simulink model "MCVT_Integration"  (R2020a)
settings: Solver=ode8  StartTime=0.0  StopTime=T_end  FixedStep=dt  RelTol=1e-3  EnableMultiTasking=on
values from neighbouring files (symbols this model uses):
  assumed_power_voltage = 1.25e2    (= 125)    [MCVT_Input_File.m]    (also in MCVT_Run_QuickCheck.m)
  battery_power_toggle = 1    [MCVT_Input_File.m]    (also in MCVT_Run_QuickCheck.m)
  battery_trickle_charge_param = 0.1    [MCVT_Input_File.m]    (also in MCVT_Run_QuickCheck.m)
  battery_trickle_leakage_param = 0.01    [MCVT_Input_File.m]    (also in MCVT_Run_QuickCheck.m)
  damage_case_id = 3    [MCVT_Input_File.m]    (also in MCVT_Run_QuickCheck.m = 1)
  dt = 1/fs    (= 0.001)    [MCVT_Run_QuickCheck.m]    (also in MCVT_Sim_Set.m)
  dt_2 = 1/fs_2    (= 0.001)    [MCVT_Run_QuickCheck.m]    (also in MCVT_Sim_Set.m)
  dt_3 = 1/fs_3    (= 0.001)    [MCVT_Run_QuickCheck.m]    (also in MCVT_Sim_Set.m)
  dt_5 = 1/fs_5    (= 0.005)    [MCVT_Run_QuickCheck.m]    (also in MCVT_Sim_Set.m)
  dt_8 = 1/fs_8    (= 0.05)    [MCVT_Run_QuickCheck.m]    (also in MCVT_Sim_Set.m)
  dt_9 = 1/fs_9    (= 0.001)    [MCVT_Run_QuickCheck.m]    (also in MCVT_Sim_Set.m)
  dust_met_duration = 3    [MCVT_Input_File.m]    (also in MCVT_Run_QuickCheck.m = 2)
  ECLSS_indoor_set_temperature = 298.15    [MCVT_Input_File.m]    (also in MCVT_Run_QuickCheck.m)
  energy_store_max_o = 150    [MCVT_Input_File.m]    (also in MCVT_Run_QuickCheck.m)
  energy_store_o = 120    [MCVT_Input_File.m]    (also in MCVT_Run_QuickCheck.m)
  fs = 1000    [MCVT_Run_QuickCheck.m]    (also in MCVT_Sim_Set.m)
  gain_charge_param = 1    [MCVT_Input_File.m]    (also in MCVT_Run_QuickCheck.m)
  ground_temperature = 373    [MCVT_Habitat_Design_Input_File.m]    (also in MCVT_Run_QuickCheck.m)
  I_max_charge = 800    [MCVT_Input_File.m]    (also in MCVT_Run_QuickCheck.m)
  I_max_discharge = 800    [MCVT_Input_File.m]    (also in MCVT_Run_QuickCheck.m)
  initial_pressure = 1e5    (= 100000)    [MCVT_Input_File.m]    (also in MCVT_Run_QuickCheck.m)
  initial_temperature = 295    [MCVT_Input_File.m]    (also in MCVT_Run_QuickCheck.m)
  interior_leak_rate = 0.00415    [MCVT_Input_File.m]    (also in MCVT_Run_QuickCheck.m)
  launch_dust_accum_start_time = 10    [MCVT_Input_File.m]    (also in MCVT_Run_QuickCheck.m = 2)
  launch_dust_accum_stop_time = 12    [MCVT_Input_File.m]    (also in MCVT_Run_QuickCheck.m = 3)
  meteorite_impact_time = 2    [MCVT_Input_File.m]    (also in MCVT_Run_QuickCheck.m = 1)
  nuclear_power_toggle = 1    [MCVT_Input_File.m]    (also in MCVT_Run_QuickCheck.m)
  print_interval = 30    [MCVT_Input_File.m]
  SOC_lower_thres = 0.5    [MCVT_Input_File.m]    (also in MCVT_Run_QuickCheck.m)
  SOC_upper_thres = 0.9    [MCVT_Input_File.m]    (also in MCVT_Run_QuickCheck.m)
  solar_power_toggle = 1    [MCVT_Input_File.m]    (also in MCVT_Run_QuickCheck.m)
  space_temperature = 4    [MCVT_Habitat_Design_Input_File.m]    (also in MCVT_Run_QuickCheck.m)
  Start_angle = 90    [MCVT_Input_File.m]    (also in MCVT_Run_QuickCheck.m)
undefined symbols (not defined in the file or in its folder): A_flow_restrictor, ACTIVITY_LOOKUP_TABLE, air, Aref, Bmap, Bmap2, Bmap3, comms, DiscIntNeverNeededParam, DmgSPL, dust_parameters, ECLSS_dust_damage_repair_time, ECLSS_heater_capacity, ECLSS_IPS_restrictor, ECLSS_IPS_setpointPressure, ECLSS_paint_damage_repair_time, ECLSS_radiatorLoop_discreteNumber, ECLSS_Vst, emptyActivity, expected_heat_add, FI, habitat, HIEM2SMM, HIEM2STM, HIEMDamageElements, impact_numbering, initial_SPLT_damage_index, input_air, input_Cond, input_CP, input_discretization, input_EV, input_Evap, input_radiatorLoop, K_ECLSS_dust_single, K_ECLSS_paint_single, K_Structure_damage_single, Kref, kW_to_W, meteoroidDustDep, mFRF, ndelays, nominalDustDep, noverlap, nuclear_toggle_gain, output_limits, panel_paint0, Ps0, reference_temperature, RepairTime_ECLSS_factor, Sensor_Location_SMM, SMM_T0, SMM_T1, SMM_T2, SMM_T3, SMM_T4, SMM_T4_0, SMM_T4_1, SMM_T4_2, solar_FDD_K ... (+73)
libraries: neural x350, simulink x39, xpcutilitieslib x7, xpcrtudplib x7, dspstat3 x1
(part 42 of 43)

== matlab function MCVT_Integration/Sys8 - Interior Environment/Habitat Interior Environment Model/AirPressureDistribution ==
  data: PrevAirPressure input; Time input; IPCS_Supply input; IPCS_Discharge input; IEN_ADJ_HIEM input; Damage input; HIEMDamageElements input; AirPressure output; IPCSAirflow output; temp0 input; pres0 input; interior_leak_rate input
function [AirPressure,IPCSAirflow] = AirPressureDistribution(PrevAirPressure,Time,IPCS_Supply,IPCS_Discharge,IEN_ADJ_HIEM,Damage,HIEMDamageElements,temp0,pres0,interior_leak_rate)
%% Documentation
% Purpose: To calculate the pressure distribution of the interior air based
%          on interactions with the STM, SMM, and ECLSS models. For
%          more details see Chapter 7 of the documentation report,
%          henceforth referred to as "report".
% Date Created: 3 June 2021
% Date Last Modified: 7 September 2021
% Modeler Name: Jeffrey Steiner (UConn)
% Funding Acknowledgement: Funded by the NASA RETHi Project (Grant #80NSSC19K1076)

% Version Number: MCVT v5

% Subsystem Connections: This code receives inputs from Subsystems 2 
%     and 5. This code ultimately sends outputs to the same subsystems, as
%     well as to Subsystem 1.

% INPUTS %
%   - PrevAirPressure: Double: previous pressure values from memory, 112x1
%       (Pa)
%   - Time: Double: Constant pertaining to simulation time used for logical
%       determinations and application of initial conditions
%   - IPCS_Supply: Double: Constant supplied by ECLSS that represents mass
%       flow of air being added to the system by the Pressure Control(kg/s)
%   - IPCS_Discharge: Double: Constant supplied by ECLSS that represents mass
%       flow of air being removed from the system by the Pressure Control(kg/s)
%   - IEN_ADJ_HIEM: Double: 112x7 matrix with columns detailing the zone 
%       number for the North, South, East, West, Top, and Bottom faces of each
%       zone, with row 1 being the number of the zone of interest
%   - Damage: Double: number of damaged nodes as given by the SMM
%   - HIEMDamageElements: Double: Vector of zone numbers corrseponding to
%       potentially damaged nodes according to mapping from the SMM
%   - temp0: Double: User-Defined initial temperature, constant (K)
%   - pres0: Double: User-Defined initial pressure, constant (K)
%   - interior_leak_rate: Double: User defined leak rate
    
% OUTPUTS %
%   - AirPressure: Double: 112x1 matrix representing the Air Pressure in
%       each corresponding zone (Pa)
%   - IPCSAirflow: Double: Constant representing discharge mass flow to the
%       ECLSS for purposes of air circulation (kg/s)

% No Function Dependencies

% No Data Dependencies

%% System Parameter Definitions
PressureDam = 0; % Exterior Environment Vacuum, Pa
NumElem = 112; % Number of finite elements
MaxIter = 1000; % Maximum number of iterations
n1 = 0.5; % Exponent for Large Openings
F = 0.83; % PowerLaw Flow Coefficient
Pinit = pres0; % Initial Pressure, Pa
Tinit = temp0; % Initial Temperature, K
PCellgsm = ones(NumElem,MaxIter)*100000; % Pressure Iteration Variable, Pa
Mu = 0.00001825;    %Air Viscosity (constant for now), kg/m-s
PCell = zeros(NumElem,2); % Pressure Reporting Variable, Pa
Rind = 287.05; %Individual Gas Constant, J/kg-K
R = 8314.41; % Universal Gas Constant, N-m/kg-mol-K
M = 28.9645; % Mean Molecular Weight per Mole, kg/kg-mole
RhoAir0 = (M*(Pinit))/(R * Tinit); %Initial Air Density, kg/m^3

%% Define Indexed Variables for Simulink

% Laminar Flow Coefficient Variables
Clf = zeros(NumElem,1);
DeltaPtrans = zeros(NumElem,1);
Ck = zeros(NumElem,1);

% Directional Mass Flows
MEast = zeros(NumElem,100);
MWest = zeros(NumElem,100);
MNorth = zeros(NumElem,100);
MSouth = zeros(NumElem,100);
MTop = zeros(NumElem,100);
MBottom = zeros(NumElem,100);

% Directional Mass Flow Derivatives
dMdPEast = zeros(NumElem,100);
dMdPWest = zeros(NumElem,100);
dMdPNorth = zeros(NumElem,100);
dMdPSouth = zeros(NumElem,100);
dMdPTop = zeros(NumElem,100);
dMdPBottom = zeros(NumElem,100);

% Iteration Variables
MCellgsm = zeros(NumElem,100);
PDeriv = zeros(NumElem,100);

% Damaged Elements as given by the SMM
Damaged = zeros(NumElem,1);

% Application of Initial Conditions
if Time == -1 || Time == 1
    PrevAirPressure = ones(NumElem,1)*Pinit;
end

if Time == -1
    for el = 1:NumElem
        PCellgsm(el,1) = Pinit;
    end
else
    PCellgsm(:,1) = PrevAirPressure;
end

%% Begin Calculation Loop

for PInc = 1

    % Damage Parameter determination, used to find leak locations
    DamagedNodes = find(Damage > 0);
    
    for checkDam = 1:length(DamagedNodes)
        Damaged(checkDam) = HIEMDamageElements(DamagedNodes(checkDam));
    end
    DamagedNZ = nonzeros(Damaged);
    DamagedElements = unique(DamagedNZ);
    
    %% System Inputs and Outputs
    
    Input1 = IPCS_Supply;
    Output1 = -(IPCS_Discharge*(Pinit/(Rind*temp0)));
    
    Inputs = [Input1];
    
    BC_SupplyFlow = Inputs(1); %kg/s
    BC_DischargeFlow = Output1; %kg/s
    
    PBC_Supply = PCellgsm(12,1) + ((BC_SupplyFlow^2)/F);
    PBC_Discharge = PCellgsm(50,1) - ((BC_DischargeFlow^2)/F);
    
    % Initiation of "While" Loop
    Condition = ones(NumElem,1);
    gsm = 1;
    
    while Condition(Condition >= 0.5)
        
        % Calculation of Mass Flows
        for Elem = 1:NumElem
            for j = 2
                if (IEN_ADJ_HIEM(j,Elem)) == 0
                    if Elem == 50
                        MEast(Elem,gsm) = F * sqrt(abs(PCellgsm(Elem,gsm) - PBC_Discharge));
                        dMdPEast(Elem,gsm) = -F * n1 / abs(PCellgsm(Elem,gsm) - PBC_Discharge);
                    else
                        MEast(Elem,gsm) = 0;
                        dMdPEast(Elem,gsm) = 0;
                    end
                    
                else
                    if PCellgsm(IEN_ADJ_HIEM(j,Elem),gsm) > PCellgsm(Elem,gsm)
                        MEast(Elem,gsm) = -F * sqrt(abs(PCellgsm(Elem,gsm) - PCellgsm(IEN_ADJ_HIEM(j,Elem),gsm)));
                        dMdPEast(Elem,gsm) = F * n1 / abs(PCellgsm(Elem,gsm) - PCellgsm(IEN_ADJ_HIEM(j,Elem),gsm));
                    elseif PCellgsm(IEN_ADJ_HIEM(j,Elem),gsm) - PCellgsm(Elem,gsm) == 0
                        dMdPEast(Elem,gsm) = 0;
                        MEast(Elem,gsm) = 0;
                    else
                        MEast(Elem,gsm) = F * sqrt(abs(PCellgsm(Elem,gsm) - PCellgsm(IEN_ADJ_HIEM(j,Elem),gsm)));
                        dMdPEast(Elem,gsm) = -F * n1 / abs(PCellgsm(Elem,gsm) - PCellgsm(IEN_ADJ_HIEM(j,Elem),gsm));
                    end
                end
            end
            for j = 3
                if (IEN_ADJ_HIEM(j,Elem)) == 0
                    if Elem == 12
                        MWest(Elem,gsm) = -F * sqrt(abs(PCellgsm(Elem,gsm) - PBC_Supply));
                        dMdPWest(Elem,gsm) = F * n1 / abs(PCellgsm(Elem,gsm) - PBC_Supply);
                    else
                        MWest(Elem,gsm) = 0;
                        dMdPWest(Elem,gsm) = 0;
                    end
                else
                    if PCellgsm(IEN_ADJ_HIEM(j,Elem),gsm) > PCellgsm(Elem,gsm)
                        MWest(Elem,gsm) = -F * sqrt(abs(PCellgsm(Elem,gsm) - PCellgsm(IEN_ADJ_HIEM(j,Elem),gsm)));
                        dMdPWest(Elem,gsm) = F * n1 / abs(PCellgsm(Elem,gsm) - PCellgsm(IEN_ADJ_HIEM(j,Elem),gsm));
                    elseif PCellgsm(IEN_ADJ_HIEM(j,Elem),gsm) - PCellgsm(Elem,gsm) == 0
                        dMdPWest(Elem,gsm) = 0;
                        MWest(Elem,gsm) = 0;
                    else
                        MWest(Elem,gsm) = F * sqrt(abs(PCellgsm(Elem,gsm) - PCellgsm(IEN_ADJ_HIEM(j,Elem),gsm)));
                        dMdPWest(Elem,gsm) = -F * n1 / abs(PCellgsm(Elem,gsm) - PCellgsm(IEN_ADJ_HIEM(j,Elem),gsm));
                    end
                end
            end
            for j = 4
                if (IEN_ADJ_HIEM(j,Elem)) == 0
                    if Elem == 1
                        MNorth(Elem,gsm) = 0;
                        dMdPNorth(Elem,gsm) = 0;
                    elseif Elem == 2
                        MNorth(Elem,gsm) = 0;
                        dMdPNorth(Elem,gsm) = 0;
                    else
                        MNorth(Elem,gsm) = 0;
                        dMdPNorth(Elem,gsm) = 0;
                    end
                else
                    if PCellgsm(IEN_ADJ_HIEM(j,Elem),gsm) > PCellgsm(Elem,gsm)
                        MNorth(Elem,gsm) = -F * sqrt(abs(PCellgsm(Elem,gsm) - PCellgsm(IEN_ADJ_HIEM(j,Elem),gsm)));
                        dMdPNorth(Elem,gsm) = F * n1 / abs(PCellgsm(Elem,gsm) - PCellgsm(IEN_ADJ_HIEM(j,Elem),gsm));
                    elseif PCellgsm(IEN_ADJ_HIEM(j,Elem),gsm) - PCellgsm(Elem,gsm) == 0
                        dMdPNorth(Elem,gsm) = 0;
                        MNorth(Elem,gsm) = 0;
                    else
                        MNorth(Elem,gsm) = F * sqrt(abs(PCellgsm(Elem,gsm) - PCellgsm(IEN_ADJ_HIEM(j,Elem),gsm)));
                        dMdPNorth(Elem,gsm) = -F * n1 / abs(PCellgsm(Elem,gsm) - PCellgsm(IEN_ADJ_HIEM(j,Elem),gsm));
                    end
                end
            end
            for j = 5
                if (IEN_ADJ_HIEM(j,Elem)) == 0
                    if Elem == 1
                        MSouth(Elem,gsm) = 0;
                        dMdPSouth(Elem,gsm) = 0;
                    elseif Elem == 2
                        MSouth(Elem,gsm) = 0;
                        dMdPSouth(Elem,gsm) = 0;
                    else
                        MSouth(Elem,gsm) = 0;
                        dMdPSouth(Elem,gsm) = 0;
                    end
                    
                else
                    if PCellgsm(IEN_ADJ_HIEM(j,Elem),gsm) > PCellgsm(Elem,gsm)
                        MSouth(Elem,gsm) = -F * sqrt(abs(PCellgsm(Elem,gsm) - PCellgsm(IEN_ADJ_HIEM(j,Elem),gsm)));
                        dMdPSouth(Elem,gsm) = F * n1 / abs(PCellgsm(Elem,gsm) - PCellgsm(IEN_ADJ_HIEM(j,Elem),gsm));
                    elseif PCellgsm(IEN_ADJ_HIEM(j,Elem),gsm) - PCellgsm(Elem,gsm) == 0
                        dMdPSouth(Elem,gsm) = 0;
                        MSouth(Elem,gsm) = 0;
                    else
                        MSouth(Elem,gsm) = F * sqrt(abs(PCellgsm(Elem,gsm) - PCellgsm(IEN_ADJ_HIEM(j,Elem),gsm)));
                        dMdPSouth(Elem,gsm) = -F * n1 / abs(PCellgsm(Elem,gsm) - PCellgsm(IEN_ADJ_HIEM(j,Elem),gsm));
                    end
                end
            end
            for j = 6
                if (IEN_ADJ_HIEM(j,Elem)) == 0
                    MTop(Elem,gsm) = 0;
                    dMdPTop(Elem,gsm) = 0;
                else
                    if PCellgsm(IEN_ADJ_HIEM(j,Elem),gsm) > PCellgsm(Elem,gsm)
                        MTop(Elem,gsm) = -F * sqrt(abs(PCellgsm(Elem,gsm) - PCellgsm(IEN_ADJ_HIEM(j,Elem),gsm)));
                        dMdPTop(Elem,gsm) = F * n1 / abs(PCellgsm(Elem,gsm) - PCellgsm(IEN_ADJ_HIEM(j,Elem),gsm));
                    elseif PCellgsm(IEN_ADJ_HIEM(j,Elem),gsm) - PCellgsm(Elem,gsm) == 0
                        dMdPTop(Elem,gsm) = 0;
                        MSouth(Elem,gsm) = 0;
                    else
                        MTop(Elem,gsm) = F * sqrt(abs(PCellgsm(Elem,gsm) - PCellgsm(IEN_ADJ_HIEM(j,Elem),gsm)));
                        dMdPTop(Elem,gsm) = -F * n1 / abs(PCellgsm(Elem,gsm) - PCellgsm(IEN_ADJ_HIEM(j,Elem),gsm));
                    end
                end
            end
            for j = 7
                if (IEN_ADJ_HIEM(j,Elem)) == 0
                    MBottom(Elem,gsm) = 0;
                    dMdPBottom(Elem,gsm) = 0;
                else
                    if PCellgsm(IEN_ADJ_HIEM(j,Elem),gsm) > PCellgsm(Elem,gsm)
                        MBottom(Elem,gsm) = -F * sqrt(abs(PCellgsm(Elem,gsm) - PCellgsm(IEN_ADJ_HIEM(j,Elem),gsm)));
                        dMdPBottom(Elem,gsm) = F * n1 / abs(PCellgsm(Elem,gsm) - PCellgsm(IEN_ADJ_HIEM(j,Elem),gsm));
                    elseif PCellgsm(IEN_ADJ_HIEM(j,Elem),gsm) - PCellgsm(Elem,gsm) == 0
                        dMdPBottom(Elem,gsm) = 0;
                        MSouth(Elem,gsm) = 0;
                    else
                        MBottom(Elem,gsm) = F * sqrt(abs(PCellgsm(Elem,gsm) - PCellgsm(IEN_ADJ_HIEM(j,Elem),gsm)));
                        dMdPBottom(Elem,gsm) = -F * n1 / abs(PCellgsm(Elem,gsm) - PCellgsm(IEN_ADJ_HIEM(j,Elem),gsm));
                    end
                end
            end
            
        end
        
        % Compilation of calculated vectors and derivatives for easy
        % code-level analysis        
        % Mass Flow Vectors
        East = MEast(:,gsm)';
        West = MWest(:,gsm)';
        North = MNorth(:,gsm)';
        South = MSouth(:,gsm)';
        Top = MTop(:,gsm)';
        Bottom = MBottom(:,gsm)';
        MassFlows = [East; West; North; South; Top; Bottom];
        % Mass Flow Derivatives
        dEast = dMdPEast(:,gsm)';
        dWest = dMdPWest(:,gsm)';
        dNorth = dMdPNorth(:,gsm)';
        dSouth = dMdPSouth(:,gsm)';
        dTop = dMdPTop(:,gsm)';
        dBottom = dMdPBottom(:,gsm)';
        dMassFlows = [dEast; dWest; dNorth; dSouth; dTop; dBottom];
        
        MCellgsm(:,gsm) = MEast(:,gsm) + MWest(:,gsm) + MNorth(:,gsm) + MSouth(:,gsm) + MTop(:,gsm) + MBottom(:,gsm);
        
        % Add in Damage components to account for leaks
        for checkDam = 1:NumElem
            for daminc = 1:length(DamagedElements)
                if checkDam == (DamagedElements(daminc))
                    BCDamFlow = interior_leak_rate * sqrt(abs(PCellgsm(checkDam,1) - PressureDam));
                    MCellgsm(checkDam,gsm) = MCellgsm(checkDam,gsm) + BCDamFlow;
                else
                    continue
                end
            end
        end
        
        PDeriv(:,gsm) = (dMdPNorth(:,gsm) + dMdPSouth(:,gsm) + dMdPWest(:,gsm) + dMdPEast(:,gsm) + dMdPTop(:,gsm) + dMdPBottom(:,gsm));
        
        % Mass Residual
        B = MCellgsm(:,gsm);
        
        dx = (32.725/NumElem)^(1/3);
        dy = (32.725/NumElem)^(1/3);
        
        % Calculation of Laminar Flow Coefficient
        Re = 30;
        Area = dx * dy;
        Dlf = (4*Area)/(4*dx);
        Ftrans = (Mu*Re*Area)/Dlf;
        
        for count = 1:NumElem
            Clf(count) = (0.6*Area)/sqrt(RhoAir0/2);
            DeltaPtrans(count) = (Ftrans/Clf(count))^2;
            Ck(count) = (Mu*Ftrans)/(RhoAir0*DeltaPtrans(count));
        end
        
        %Assemble Jacobian Matrix
        JCB = zeros(NumElem);
        
        for JCBi = 1:NumElem
            
            A=IEN_ADJ_HIEM(2:end,JCBi);
            NZ=A(A~=0);
            for k = 1:NumElem
                if k==JCBi
                    JCB(JCBi,k) = 1;
                elseif k == NZ(NZ == k)
                    if k < JCBi
                        JCB(JCBi,k) = JCB(k,JCBi);
                    else
                        if (PCellgsm(k,gsm)-PCellgsm(JCBi,gsm)) == 0
                            JCB(JCBi,k) = F * n1 * (0.0001^-n1);
                        else
                            JCB(JCBi,k) = ((-F * n1)/sqrt(abs((PCellgsm(k,gsm)-PCellgsm(JCBi,gsm)))));
                        end
                    end
                else
                    JCB(JCBi,k) = 0;
                end
            end
            if JCBi == 12
                DiagSum = [1:(JCBi-1) (JCBi+1):NumElem];
                JCB(JCBi,JCBi) = sum(abs(JCB(JCBi,DiagSum))) + abs(((-F * n1)/sqrt(abs((PCellgsm(JCBi,gsm)-PBC_Supply)))));

            elseif JCBi == 50
                DiagSum = [1:(JCBi-1) (JCBi+1):NumElem];
                JCB(JCBi,JCBi) = sum(abs(JCB(JCBi,DiagSum))) + abs(((-F * n1)/sqrt(abs((PCellgsm(JCBi,gsm)-PBC_Discharge)))));
            else
                if JCBi == 1
                    DiagSum = [(JCBi+1):NumElem];
                else
                    DiagSum = [1:(JCBi-1) (JCBi+1):NumElem];
                end
                JCB(JCBi,JCBi) = sum(abs(JCB(JCBi,DiagSum)));
            end
        end
        
        % Determine Pressure Correction Vector
        C = zeros(NumElem,1);
        C(:,1) = B'/JCB;
        
        % Update Pressure Vector
        PCellgsm(:,gsm+1) = PCellgsm(:,gsm) - C;
        
        % Establish value of convergence check
        Condition = abs(PCellgsm(:,gsm+1)-PCellgsm(:,gsm));
        
        % Increase increment value
        gsm = gsm+1;
        
    end
    
    % Override to prevent negative pressure values in case of pressure
    % reductions
    if PCellgsm(PCellgsm < 0) < 0
        PCellgsm(PCellgsm < 0) = 0;
    end
    
    % Update pressure recording variable
    PCell(:,PInc+1) = PCellgsm(:,gsm);
end

%% Output Initialization and Execution

AirPressure = squeeze(PCell(:,2)); % Air Pressure Distribution, Pa
IPCSAirflow = IPCS_Discharge; % Airflow to ECLSS, kg/s

end

== matlab function MCVT_Integration/Sys8 - Interior Environment/Habitat Interior Environment Model/AirTemperatureDistribution ==
  data: PCell0 input; HIEMDamageElements input; RefTemp input; pres0 input; temp0 input; Damage input; HCAirFlow input; WallTemp input; AirTemp output; ThermalManHeatingInp input; ThermalManHeatingInpLoc input; ThermalManCoolingInp input; ThermalManCoolingInpLoc input; AirTempWall output; Time input; PSTemp output size=1; dt input; IEN_ADJ_HIEM input; HumanHeat input; HumanElement input; TMSAirflow output; air_specific_heat input; habitat_volume input
function [AirTemp,AirTempWall,PSTemp,TMSAirflow] = TemperatureDistribution3D(PCell0,HIEMDamageElements,RefTemp,pres0,temp0,Damage,HCAirFlow,WallTemp,ThermalManHeatingInp,ThermalManHeatingInpLoc,ThermalManCoolingInp,ThermalManCoolingInpLoc,Time,dt,IEN_ADJ_HIEM,HumanHeat,HumanElement,air_specific_heat,habitat_volume)
%% Documentation
% Purpose: To calculate the temperature distribution of the interior air based
%          on interactions with the STM, SMM, Human, and ECLSS models. For
%          more details see Chapter 7 of the documentation report,
%          henceforth referred to as "report".
% Date Created: 3 June 2021
% Date Last Modified: 7 September 2021
% Modeler Name: Jeffrey Steiner (UConn)
% Funding Acknowledgement: Funded by the NASA RETHi Project (Grant #80NSSC19K1076)

% Version Number: MCVT v5

% Subsystem Connections: This code receives inputs from Subsystems 2, 5, 6,
%     and 9. This code sends outputs to Subsystems 1, 2, 5, and 6.

% INPUTS %
%   - PCell0: Double: Pressure Distribution, Pa
%   - HIEMDamageElements: Double: Vector of zone numbers corresponding to
%       potentially damaged nodes according to mapping from the SMM
%   - RefTemp: Double: User-Defined Reference Temperature for the Exterior
%   Environment in case of leak scenario (K)
%   - pres0: Double: User-Defined initial pressure, constant (Pa)
%   - temp0: Double: User-Defined initial temperature, constant (K)
%   - Damage: Double: number of damaged nodes as given by the SMM
%   - HCAirFlow: Double: Constant supplied by ECLSS that represents mass
%       flow of air being added to the system from the temperature control(kg/s)
%   - WallTemp: Double: Mapped interior wall surface temperature from STM,
%       64x1 (K)
%   - ThermalManHeatingInp: Double: Constant Total Heating Energy Input 
%     from ECLSS Thermal Management (J) 
%   - ThermalManHeatingInpLoc: Double: Zone number corresponding to heating
%       input location for ECLSS Thermal Management
%   - ThermalManCoolingInp: Double: Constant Cooling Temperature Setpoint
%     Input from ECLSS Thermal Management (K) 
%   - ThermalManCoolingInpLoc: Double: Zone number corresponding to cooling
%       input location for ECLSS Thermal Management
%   - Time: Double: Constant pertaining to simulation time used for logical
%       determinations and application of initial conditions
%   - dt: Double: constant representing the time step used for the model
%       (s)
%   - IEN_ADJ_HIEM: Double: 112x7 matrix with columns detailing the zone 
%       number for the North, South, East, West, Top, and Bottom faces of each
%       zone, with row 1 being the number of the zone of interest
%   - HumanHeat: Double: Constant Total Heating Energy Input from Human 
%       Agent Model (J) 
%   - HumanElement: Double: Zone number corresponding to heating input
%       location for Human Agent Model
%   - air_specific_heat: Double: Specific Heat of Air, J/kg-K
%   - habitat_volume: Double: User-defined habitat volume, m^3
    
% OUTPUTS %
%   - AirTemp: Double: 112x1 matrix representing the Air Temperature in
%       each corresponding zone (K)
%   - AirTempWall: 20x1 matrix corresponding to the Air Temperature in
%       zones in contact with the structural wall (K), sent to STM
%   - PSTemp: Double: Constant Zonal Temperature corresponding to zone in
%       which ECLSS Cooling input is provided (K), provided to ECLSS Thermal
%       Management
%   - TMSAirflow: Double: Constant representing discharge mass flow to the
%       ECLSS Thermal Management for purposes of air circulation (kg/s)

% No Function Dependencies

% No Data Dependencies

%% System Parameter Definitions

NumElem = length(IEN_ADJ_HIEM); % Number of Elements in the system
TCell0 = temp0; %Initial Air Temperature, K
Rind = 287.05; %Individual Gas Constant, J/kg-K
WallElements = [1;2;3;4;5;6;7;8;9;10;11;12;13;14;15;16;29;30;31;32;45;46;47;48;49;50;51;52;53;54;55;56;57;58;59;60;61;62;75;76;77;78;79;80;81;82;83;84;97;98;99;100;101;102;103;104;105;106;107;108;109;110;111;112];
h = 10;
WallElemArea = ((habitat_volume * 3)/2.5)/length(WallElements);
TBS = 293.15;
%% Define Indexed Variables for Simulink

% Directional Energy Flows
QEast = zeros(NumElem,1);
QWest = zeros(NumElem,1);
QNorth = zeros(NumElem,1);
QSouth = zeros(NumElem,1);
QTop = zeros(NumElem,1);
QBottom = zeros(NumElem,1);
QCell = zeros(NumElem,1);

RhoAir0 = pres0/(Rind * temp0); %Initial Air Density, kg/m^3

% Heat Conduction Coefficient
Sys8_CondCoeff = zeros(NumElem,1);

% Heat Change Iteration Variable
dTCell = zeros(NumElem,1);

% Damaged Elements as given by the SMM
Damaged = zeros(NumElem,1);

% Temeprature Recording Variable
TCell = ones(NumElem,2);

if Time == -1
    for el = 1:NumElem
        TCell(el,1) = TCell0;
    end
    MassFlows = zeros(6,NumElem);
else
    TCell(:,1) = PCell0(:,1)/(RhoAir0*Rind);
end

%% Definition of Wall Boundary Condition

% Set Cooling input to 0 if no airflow is provided
if HCAirFlow == 0
    ThermalManCoolingInp = 0;
end

%% Temperature Calculation Loop Initialization

% Zonal Density Variable
RhoAir0 = zeros(NumElem,1);
RhoAir0(:,1) = PCell0(:,1)/(Rind * TCell0); %Initial Air Density, kg/m^3

Sys8_AirCond = 0.025; %Thermal Conductivity of air, W/m-K
Sys8_CondCoeff(:,1) = (Sys8_AirCond./(RhoAir0(:,1)*air_specific_heat)); % Thermal Conduction Coefficient

% Loop calculating temperature change from Heating/Cooling as received by
% ECLSS, K
if HCAirFlow == 0
    CoolingInp = 0;
    HeatingInp = ((ThermalManHeatingInp/(air_specific_heat * RhoAir0(ThermalManHeatingInpLoc,1) * 1))*dt);
else
    CoolingInp = (air_specific_heat * (HCAirFlow * RhoAir0(ThermalManCoolingInpLoc,1)) * (ThermalManCoolingInp - TCell(ThermalManCoolingInpLoc,1))/(air_specific_heat * RhoAir0(ThermalManCoolingInpLoc,1) * 1))*dt;
    HeatingInp = ((ThermalManHeatingInp/(air_specific_heat * RhoAir0(ThermalManHeatingInpLoc,1) * 1))*dt);
end

%% Begin Calculation Loop

for Time = 1
    %% System Inputs and Outputs
    
    % Initiation of "While" Loop
    Condition = ones(NumElem,1);
    
    while Condition(Condition >= 0.5)
        WallElementCount = 1;
        % Calculation of Directional Energy Balances
        for Elem = 1:NumElem
            for j = 2
                if (IEN_ADJ_HIEM(j,Elem)) == 0
                    QEast(Elem,1) = (((Sys8_CondCoeff(Elem,1) * (WallTemp(WallElementCount,1) - TCell(Elem,1)))*(air_specific_heat * RhoAir0(Elem,1) * (sqrt(WallElemArea)/2))) + ((h * WallElemArea * (WallTemp(WallElementCount,1) - TCell(Elem,1)))/(air_specific_heat * RhoAir0(Elem,1) * (habitat_volume/NumElem))));
                end
            end
            for j = 3
                if (IEN_ADJ_HIEM(j,Elem)) == 0
                    QWest(Elem,1) = (((Sys8_CondCoeff(Elem,1) * (WallTemp(WallElementCount,1) - TCell(Elem,1)))*(air_specific_heat * RhoAir0(Elem,1) * (sqrt(WallElemArea)/2))) + ((h * WallElemArea * (WallTemp(WallElementCount,1) - TCell(Elem,1)))/(air_specific_heat * RhoAir0(Elem,1) * (habitat_volume/NumElem))));
                end
            end
            for j = 4
                if (IEN_ADJ_HIEM(j,Elem)) == 0
                    QNorth(Elem,1) = (((Sys8_CondCoeff(Elem,1) * (WallTemp(WallElementCount,1) - TCell(Elem,1)))*(air_specific_heat * RhoAir0(Elem,1) * (sqrt(WallElemArea)/2))) + ((h * WallElemArea * (WallTemp(WallElementCount,1) - TCell(Elem,1)))/(air_specific_heat * RhoAir0(Elem,1) * (habitat_volume/NumElem))));
                end
            end
            for j = 5
                if (IEN_ADJ_HIEM(j,Elem)) == 0
                    QSouth(Elem,1) = (((Sys8_CondCoeff(Elem,1) * (WallTemp(WallElementCount,1) - TCell(Elem,1)))*(air_specific_heat * RhoAir0(Elem,1) * (sqrt(WallElemArea)/2))) + ((h * WallElemArea * (WallTemp(WallElementCount,1) - TCell(Elem,1)))/(air_specific_heat * RhoAir0(Elem,1) * (habitat_volume/NumElem))));
                end
            end
            for j = 6
                if (IEN_ADJ_HIEM(j,Elem)) == 0
                    QTop(Elem,1) = (((Sys8_CondCoeff(Elem,1) * (WallTemp(WallElementCount,1) - TCell(Elem,1)))*(air_specific_heat * RhoAir0(Elem,1) * (sqrt(WallElemArea)/2))) + ((h * WallElemArea * (WallTemp(WallElementCount,1) - TCell(Elem,1)))/(air_specific_heat * RhoAir0(Elem,1) * (habitat_volume/NumElem))));
                end
            end
            for j = 7
                if (IEN_ADJ_HIEM(j,Elem)) == 0
                    QBottom(Elem,1) = (((Sys8_CondCoeff(Elem,1) * (TBS - TCell(Elem,1)))*(air_specific_heat * RhoAir0(Elem,1) * (sqrt(WallElemArea)/2))) + ((h * WallElemArea * (TBS - TCell(Elem,1)))/(air_specific_heat * RhoAir0(Elem,1) * (habitat_volume/NumElem))));
                end
            end
            
            % Increase wall element count - used for sequential application
            % of wall temperature from STM
            if IEN_ADJ_HIEM(IEN_ADJ_HIEM(2:6,Elem) == 0)
                WallElementCount = WallElementCount + 1;
            end
        end
        
        % Compilation of calculated vectors for easy code-level analysis
        % Energy Flow Vectors
        East = QEast(:,1)';
        West = QWest(:,1)';
        North = QNorth(:,1)';
        South = QSouth(:,1)';
        Top = QTop(:,1)';
        Bottom = QBottom(:,1)';
        EnergyFlows = [East; West; North; South; Top; Bottom];
        
        QCell(:,1) = QEast(:,1) + QWest(:,1) + QNorth(:,1) + QSouth(:,1) + QTop(:,1) + QBottom(:,1);
        
        % Consider ECLSS Input
        
        QCell(ThermalManHeatingInpLoc,1) = QCell(ThermalManHeatingInpLoc,1) + HeatingInp;
        QCell(ThermalManCoolingInpLoc,1) = QCell(ThermalManCoolingInpLoc,1) + CoolingInp;
        
        % Consider Damage Input
        
        DamagedNodes = find(Damage > 0);
        
        for checkDam = 1:length(DamagedNodes)
            Damaged(checkDam) = HIEMDamageElements(DamagedNodes(checkDam));
        end
        DamagedElements = unique(Damaged);
        
        for checkDam = 1:NumElem
            for daminc = 1:length(DamagedElements)
                if checkDam == (DamagedElements(daminc))
                    BCDamHeat = ((-h * WallElemArea * (TCell(checkDam,1) - RefTemp))/(air_specific_heat * RhoAir0(checkDam,1) * (habitat_volume/NumElem)));
                    QCell(checkDam,1) = QCell(checkDam,1) + BCDamHeat;
                else
                    continue
                end
            end
        end
        
        % Determine temperature change
        for Sys8_TempInc = 1:NumElem
            dTCell(Sys8_TempInc,1) = (QCell(Sys8_TempInc,1)*dt)/((RhoAir0(Sys8_TempInc,1)*(habitat_volume/NumElem)) * air_specific_heat);
        end
        
        % Consider Human Input, (Given as a value of Kelvin)
        
        dTCell(HumanElement,1) = dTCell(HumanElement,1) + HumanHeat;
        
        % Apply temperature change
        TCell(:,2) = TCell(:,1) + dTCell(:,1);
        
        % Update convergence condition
        Condition = abs(TCell(:,2) - TCell(:,1));
        
        % Update temperature recording variable for next iteration
        TCell(:,1) = TCell(:,2);
    end    
end
%% Output Initialization and Execution

% Outputs to memory blocks
AirTemp = TCell(:,2);

% Designation of Wall-Adjacent Element Temperature Output
OuterElements = zeros(64,1);
ATW = zeros(64,1);
count = 1;
for check = 1:NumElem
    if IEN_ADJ_HIEM(2,check) == 0
        OuterElements(count) = IEN_ADJ_HIEM(1,check);
        ATW(count) = AirTemp(OuterElements(count),1);
        count = count + 1;
    
    elseif IEN_ADJ_HIEM(3,check) == 0
        OuterElements(count) = IEN_ADJ_HIEM(1,check);
        ATW(count) = AirTemp(OuterElements(count),1);
        count = count + 1;
    
    elseif IEN_ADJ_HIEM(4,check) == 0
        OuterElements(count) = IEN_ADJ_HIEM(1,check);
        ATW(count) = AirTemp(OuterElements(count),1);
        count = count + 1;
    
    elseif IEN_ADJ_HIEM(5,check) == 0
        OuterElements(count) = IEN_ADJ_HIEM(1,check);
        ATW(count) = AirTemp(OuterElements(count),1);
        count = count + 1;
    
    elseif IEN_ADJ_HIEM(6,check) == 0
        OuterElements(count) = IEN_ADJ_HIEM(1,check);
        ATW(count) = AirTemp(OuterElements(count),1);
        count = count + 1;
    end
end

% Final Outputs
TMSAirflow = HCAirFlow; % Airflow Output to ECLSS (Constant)
AirTempWall = ATW(:,1); % Wall-Adjacent temp output (to STM), K
PSTemp = TCell(ThermalManCoolingInpLoc,2); % Reference Temp for ECLSS, K

end

== matlab function MCVT_Integration/Sys2 - Structural System/Sys2_Structural_System/Structural Thermal Model 2.2/STM Temperature Sensors/ Resistance Temperature Detector1/Switch ON//OFF Control Block/Switch OFF/MATLAB Function1 ==
  data: u input; y output; Time input
function y = fcn(u,Time)

if Time>=0
    u = NaN;
end

y = u;

== matlab function MCVT_Integration/Sys5 - ECLSS/Active Thermal Control System (ATCS)/MATLAB Function6 ==
  data: P_request output; Ps input; lim_supply input; Ph output; Qh input
function [ P_request, Ph] = heater_capacity(Ps, lim_supply, Qh )
%% Documentation
% Purpose: Output heating power and power request for next timestep
% Date Created: 20 June 2020
% Date Last Modified: 27 July 2021
% Modeler Name: Jaewon Park (Purdue)
% Funding Acknowledgement: Funded by the NASA RETHi Project (80NSSC19K1076)

% Version Number: MCVT v1.4

% Subsystem Connections: receives input from subsystem 3 and sends output to subsystem 8.

%%% All inputs and outputs to this function are 1 dimensional %%%
% INPUTS %
%   - Ps: power required for heating, unit: kW, type: float
%   - Qh: heating capacity, unit: kW, type: float
%   - lim_supply: limited power supply, unit: kW, type: float
    
% OUTPUTS %
%   - Ph: heating power, unit: kW, type: float
%   - P_request: power supply request, unit: kW, type: float

% Function Dependencies: No dependency

% No Data Depenencies
%% Calculated power
Ph = min([Ps,Qh,lim_supply]);   % output heating power is the minimum of three inputs
P_request = min([Ps,Qh]);       % it will also request power it would have needed 

end

== matlab function MCVT_Integration/Sys5 - ECLSS/Active Thermal Control System (ATCS)/Sensors ==
  data: Tfs input; TTfs output
function TTfs= sensors_HTF(Tfs)
%% Documentation
% Purpose: Simulate sensor errors
% Date Created: 20 June 2020
% Date Last Modified: 27 July 2021
% Modeler Name: Jaewon Park, CJ Pan (Purdue)
% Funding Acknowledgement: Funded by the NASA RETHi Project (80NSSC19K1076)

% Version Number: MCVT v1.4

% Subsystem Connections: No connection.

% INPUTS %
%   - Tfs: temperature reading, unit: C, type: float
    
% OUTPUTS %
%   - TTfs: temperature reading with noise, unit: C, type: float

% Function Dependencies: No dependency

% No Data Depenencies
%% adding noise (to do)
N=length(Tfs);

n=zeros(1,N);


for i=1:N
    n(i)=normrnd(0,0.1);
end

TTfs=Tfs+n;


end

== matlab function MCVT_Integration/Sys5 - ECLSS/Active Thermal Control System (ATCS)/Sensors1 ==
  data: Trs input; TTrs output
function TTrs= sensors_rfin(Trs)
%% Documentation
% Purpose: Simulate sensor errors
% Date Created: 20 June 2020
% Date Last Modified: 27 July 2021
% Modeler Name: Jaewon Park, CJ Pan (Purdue)
% Funding Acknowledgement: Funded by the NASA RETHi Project (80NSSC19K1076)

% Version Number: MCVT v1.4

% Subsystem Connections: No connection.

% INPUTS %
%   - Tfs: temperature reading, unit: C, type: float
    
% OUTPUTS %
%   - TTfs: temperature reading with noise, unit: C, type: float

% Function Dependencies: No dependency

% No Data Depenencies
%% adding noise (to do)

N=length(Trs);

n=zeros(1,N);


for i=1:N
    n(i)=normrnd(0,0.1);
end

TTrs=Trs+n;


end

== matlab function MCVT_Integration/Sys5 - ECLSS/Active Thermal Control System (ATCS)/Variable set temperature ==
  data: Pd input; Tset0 input; Ps input; Tset output; Tset input; set input; set output
function [Tset ,set] =set_temperature_change(Pd, Tset0, Ps,  Tset, set)
%% Documentation
% Purpose: Variable temperature setpoint adjustment. When there is less
% power available than the amount needed, setpoint temperature is raised little bit 
% so that refridgerant cycle consume less power. This only works in cooling
% scenario (when interior environment needs cooling).
% Date Created: 20 June 2020
% Date Last Modified: 30 Aug 2021
% Modeler Name: Jaewon Park, CJ Pan (Purdue)
% Funding Acknowledgement: Funded by the NASA RETHi Project (80NSSC19K1076)

% Version Number: MCVT v1.4

% Subsystem Connections: receives input from subsystem 3.

%%% All inputs and outputs to this function are 1 dimensional %%%
% INPUTS %
%   - Tset0: initial temperature setpoint, unit: C, type: float
%   - dP_upper: power upper threshold, unit: kW, type: float
%   - dP_lower: power lower threshold, unit: kW, type: float
%   - Ps: power supply, unit: kW, type: float
%   - Pd: power demand, unit: kW, type: float
%   - Tset: current setpoint temperature, unit: C, type: float
%   - set: temperature setting indicator, type: logical
    
% OUTPUTS %
%   - Tset: current setpoint temperature, unit: C, type: float
%   - set: stemperature setting indicator, type: logical

% Function Dependencies: No dependency

% No Data Depenencies

%% Setpoint temperature adjustment
bandwidth_for_control = 0.0000001;      % bandwidth for control. The number is selected based on trial and error. 
dP = bandwidth_for_control*Ps;          % setting limit (to upper and lower bound) to adjust controller sensitivity
if Pd>=(Ps+dP)                          % if power demand is larger than what's supplied (and a bit more)
    set=1;                              % temperature setpoint needs to be adjusted 
elseif Pd<=(Ps-dP)                      % if power demand is less than what's supplied (and a bit less)
    set=0;                              % temperature setpoint needs no adjustment
end

if set==1                               % if setpoint temperature needs to be adjusted     
    Tset=Tset+0.1*(Pd-Ps);              % raise the setpoint temperature a bit depending on how much more energy is needed from supply
else                                    % if no need for setpoint adjustment
    if Tset>Tset0                       % if setpoint has been adjusted before
    Tset=Tset-0.1*(Ps-Pd);              % lower the setpoint temperature a bit depending on how much energy is oversupplied
    else                                % in the case that setpoint temperature has been lowered to far 
        Tset=Tset0;                     % bring the setpoint temperature to initial setpoint 
    end
end

end

== matlab function MCVT_Integration/Sys5 - ECLSS/Active Thermal Control System (ATCS)/Vavle opening control ==
  data: Ye input; input_EV output; Ye_set input; input_EV input
function input_EV= EV_control(Ye, Ye_set, input_EV)
%% Documentation
% Purpose: empirical control of expansion valve
% Date Created: 20 June 2020
% Date Last Modified: 27 July 2021
% Modeler Name: Jaewon Park, CJ Pan (Purdue)
% Funding Acknowledgement: Funded by the NASA RETHi Project (80NSSC19K1076)

% Version Number: MCVT v1.4

% Subsystem Connections: No connection.

% INPUTS %
%   - Ye: liquid volume fraction of evaporator, type: float
%   - Ye_set: liquid volume fraction of evaporator setpoint, type: float
%   - input_EV: initial input parameter for expansion valve, [1x2]
    
% OUTPUTS %
%   - input_EV: new expansion valve parameter, [1x2]

% Function Dependencies: No dependency

% No Data Depenencies
%% expansion valve control
a1=(Ye-Ye_set)^2;
a2=Ye_set-Ye;
aEV=0.05+20*a1+20*a2;   % coefficients are chosen based on iteration, there can be other values with which the controller works fine. 
        
input_EV(2)=aEV; % adjusting valve opening of expansion valve 


end

== matlab function MCVT_Integration/Sys5 - ECLSS/Interior Pressure Control System (IPCS)/High pressure stored air ==
  data: Psk input; dt input; Ps output; ms input; ECLSS_Vst input
function Ps = Air_storage_tank(Psk,dt, ms, ECLSS_Vst)
%% Documentation
% Purpose: Find air storage tank pressure
% Date Created: 20 June 2020
% Date Last Modified: 1 Sept 2021
% Modeler Name: Jaewon Park, CJ Pan (Purdue)
% Funding Acknowledgement: Funded by the NASA RETHi Project (80NSSC19K1076)

% Version Number: MCVT v1.4

% Subsystem Connections: No connection.

%%% All inputs and outputs to this function are 1 dimensional %%%
% INPUTS %
%   - Psk: tank pressure at the previous time step, unit: Pa, type: float
%   - dt: time step (dt_5 for ECLSS), unit: s, type: float
%   - ms: mass flow rate of supplied air, unit: kg/s, type: float
%   - ECLSS_Vst: storage tank volume, unit: m^3, type: float
    
% OUTPUTS %
%   - Ps: tank pressure at the current time step, unit: Pa, type: float

% Function Dependencies: No dependency.

% No Data Depenencies
%% Air storage tank pressure calculation 
drou_dP = SVR_drou_air(Psk);        % find drho/dP of storage 
Ps = -ms*dt/ECLSS_Vst/drou_dP+Psk;  % find tank pressure 

end

function fval=SVR_drou_air(x)
%% Documentation
% Purpose: Find property of air. This function especially tries to find 
%           change of air density per change of air pressure at the given 
%           air pressure. It is a numerical differentiation from tabulated
%           air property data. 
% Date Created: 20 June 2020
% Date Last Modified: 1 Sept 2021
% Modeler Name: Jaewon Park, CJ Pan (Purdue)

% Version Number: MCVT v1.4

% Subsystem Connections: No connection.

%%% All inputs and outputs to this function are 1 dimensional %%%
% INPUTS %
%   - x: pressure, unit: bar, type: float
    
% OUTPUTS %
%   - fval: saturated enthalpy, unit: kJ/kg, type: float

% Function Dependencies: This function is called by Air_storage_tank
% This function calls following subfunction
% RBF_kernel_drou_air

% No Data Depenencies
%% Data for fitting
% data obtained from https://webbook.nist.gov/chemistry/ for air (nitrogen)
 b0=9.8620;                                                                 % bias term for regression
 U=[1.97014035323934,1.05426362522981,0.323664232192873,-0.187384918684283,...
     -0.470144611371594,-0.541755273517672,-0.441957635398190,...
     -0.226622291743171,0.0409342364298861,0.299477977133928,...
     0.498701470770185,0.605962480198090,0.609859309132522,...
     0.520137708349258,0.364066552606112,0.180031696559702,...
     -0.110719878310510,-0.116918137761999,0.166367798111747,...
     0.526487671185185,0.698404311261932,0.558499701573089,...
     0.210636486603980,-0.0920389641597414,-0.131679880060262,...
     0.113423773900682,0.445787466457089,0.584379879643995,...
     0.370369122149642,-0.0993042507137863,-0.512102371835571,...
     -0.542538554355633,-0.0933406074869467,0.584507475685357,...
     0.982838016295136,0.596540523275546,-0.796187708452593,...
     -1.82310925233934];                                                    % stored training output of regression model
 XX=[0.6;1.1;1.6;2.1;2.6;3.1;3.6;4.1;4.6;5.1;5.6;6.1;6.6;7.1;7.6;8.1;9.1;...
     10.1; 11.1; 12.1;13.1000000000000;14.1000000000000;15.1000000000000;...
     16.1000000000000;17.1000000000000;18.1000000000000;19.1000000000000;...
     20.1000000000000;21.1000000000000;22.1000000000000;23.1000000000000;...
     24.1000000000000;25.1000000000000;26.1000000000000;27.1000000000000;...
     28.1000000000000;29.1000000000000;29.6000000000000];                   % stored training input of regression model 

%% Calculate drho/dP of air 
K=RBF_kernel_drou_air(x,XX');                       % get kernal value based on radial basis function
fval=K*U'+b0;                                       % evaluate the drho/dP
end

%% Regression by RBF 
function K=RBF_kernel_drou_air(A,B)
%% Documentation
% Purpose: Calculate Gaussian Kernel based on Radial Basis Function (RBF) 
% Date Created: 20 June 2020
% Date Last Modified: 1 Sept 2021
% Modeler Name: Jaewon Park, CJ Pan (Purdue)

% Version Number: MCVT v1.4

% Subsystem Connections: No connection.

% INPUTS %
%   - A: query point, unit: unitless, type: float, 1-D
%   - B: training data input, unit: unitless, type: float, 2-D vector (length of the vector does not matter)  

% OUTPUTS %
%   - K: Kernal value, unit: C, type: float, 2-D vector of same size as the
%   input B

% Function Dependencies: This function is called by SVR_drou_air

    delta=4.0;                              % length scale for kernel smoother. This is manually set so that the regression works well. 
    r=abs(A'-B);                            % following the formula for Gaussian Kernel Smoother
    K=exp(-r.^2./(2*delta^2));
end

== matlab function MCVT_Integration/Sys5 - ECLSS/Interior Pressure Control System (IPCS)/Power consumption Calculator ==
  data: Fr_now input; Fr_100 input; Rel_now input; Rel_100 input; power output; ECLSS_IPS_valve parameter
function power = power_comsumption(Fr_now,Fr_100, Rel_now, Rel_100, ECLSS_IPS_valve)
%% Documentation
% Purpose: Find power consumption by the valves
% Date Created: 20 June 2020
% Date Last Modified: 27 July 2021
% Modeler Name: Jaewon Park (Purdue)
% Funding Acknowledgement: Funded by the NASA RETHi Project (80NSSC19K1076)

% Version Number: MCVT v1.4

% Subsystem Connections: sends output to subsystem 3.

%%% All inputs and outputs to this function are 1 dimensional %%%
% INPUTS %
%   - Fr_now: effective cross-sectional area for flow restrictor, unit: m^2, type: float
%   - Fr_100: maximum possible cross-sectional area for flow restrictor, unit: m^2, type: float
%   - Rel_now: cross-sectional area for relief valve, unit: m^2, type: float
%   - Rel_100: maximum possible cross-sectional area for relief valve, unit: m^2, type: float
%   - ECLSS_IPS_valve: valve power consumption, type: struct (see below for dot indexing)
%                       max power: control valve power consumption when fully opened, unit: W
%                       min power: control valve power consumption when fully closed, unit: W

% OUTPUTS %
%   - power: total power consumed by the valves, unit: kW, type: float

% Function Dependencies: No dependency  

% No Data Depenencies
%%
convert_kW2W = 1000;                                        % unit conversion from kW to W
p_Fr = (Fr_now/Fr_100) * (ECLSS_IPS_valve.max_power-ECLSS_IPS_valve.min_power)...
    + ECLSS_IPS_valve.min_power;                            % power consumed by valve between storage tank and flow restrictor, unit: W
p_Rel = (Rel_now/Rel_100) * (ECLSS_IPS_valve.max_power-ECLSS_IPS_valve.min_power)...
    + ECLSS_IPS_valve.min_power;                            % power consumed by relief valve, unit: W
power = (p_Fr + p_Rel)/convert_kW2W;                        % sum of the two power above; total power consumed by the valves, unit: kW

end

== matlab function MCVT_Integration/Sys5 - ECLSS/Interior Pressure Control System (IPCS)/Relief Valve Control ==
  data: Aref input; Kref input; Pint input; m_rel output; air parameter
function m_rel = relief_valve_control(Aref, Kref, Pint, air)
%% Documentation
% Purpose: Find mass flow rate of the air released through the relief valve
% Date Created: 20 June 2020
% Date Last Modified: 27 July 2021
% Modeler Name: Jaewon Park, CJ Pan (Purdue)
% Funding Acknowledgement: Funded by the NASA RETHi Project (80NSSC19K1076)

% Version Number: MCVT v1.4

% Subsystem Connections: Sends output to subsystem 8.

%%% All inputs and outputs to this function are 1 dimensional %%%
% INPUTS %
%   - rho_air: density of air, unit: kg/m^3, type: float
%   - Aref: effective cross-sectional area of relief valve, unit: m^2, type: float
%   - Kref: valve coefficient of relief valve, unit: m^3/(s*Pa^0.5), type: float
%   - Pint: pressure of interior habitat, unit: Pa, type: float
    
% OUTPUTS %
%   - m_rel: mass flow rate of relief valve, unit: kg/s, type: float

% Function Dependencies: No dependency

% No Data Depenencies
%% Valve opening decision
% if there is a pressure gradient between the interior habitat and space 
if Pint > 0
    m_rel = -Kref * Aref * air.density * sqrt(Pint);  % mass flow rate from relief valve
% for the case where interior habitat pressure is zero of below (vacuum)
else
    m_rel = 0;                              % no flow is allowed 
end
